simulink model "MCVT_Integration"  (R2020a)
settings: Solver=ode8  StartTime=0.0  StopTime=T_end  FixedStep=dt  RelTol=1e-3  EnableMultiTasking=on
values from neighbouring files (symbols this model uses):
  assumed_power_voltage = 1.25e2    (= 125)    [MCVT_Input_File.m]    (also in MCVT_Run_QuickCheck.m)
  battery_power_toggle = 1    [MCVT_Input_File.m]    (also in MCVT_Run_QuickCheck.m)
  battery_trickle_charge_param = 0.1    [MCVT_Input_File.m]    (also in MCVT_Run_QuickCheck.m)
  battery_trickle_leakage_param = 0.01    [MCVT_Input_File.m]    (also in MCVT_Run_QuickCheck.m)
  damage_case_id = 3    [MCVT_Input_File.m]    (also in MCVT_Run_QuickCheck.m = 1)
  dt = 1/fs    (= 0.001)    [MCVT_Run_QuickCheck.m]    (also in MCVT_Sim_Set.m)
  dt_2 = 1/fs_2    (= 0.001)    [MCVT_Run_QuickCheck.m]    (also in MCVT_Sim_Set.m)
  dt_3 = 1/fs_3    (= 0.001)    [MCVT_Run_QuickCheck.m]    (also in MCVT_Sim_Set.m)
  dt_5 = 1/fs_5    (= 0.005)    [MCVT_Run_QuickCheck.m]    (also in MCVT_Sim_Set.m)
  dt_8 = 1/fs_8    (= 0.05)    [MCVT_Run_QuickCheck.m]    (also in MCVT_Sim_Set.m)
  dt_9 = 1/fs_9    (= 0.001)    [MCVT_Run_QuickCheck.m]    (also in MCVT_Sim_Set.m)
  dust_met_duration = 3    [MCVT_Input_File.m]    (also in MCVT_Run_QuickCheck.m = 2)
  ECLSS_indoor_set_temperature = 298.15    [MCVT_Input_File.m]    (also in MCVT_Run_QuickCheck.m)
  energy_store_max_o = 150    [MCVT_Input_File.m]    (also in MCVT_Run_QuickCheck.m)
  energy_store_o = 120    [MCVT_Input_File.m]    (also in MCVT_Run_QuickCheck.m)
  fs = 1000    [MCVT_Run_QuickCheck.m]    (also in MCVT_Sim_Set.m)
  gain_charge_param = 1    [MCVT_Input_File.m]    (also in MCVT_Run_QuickCheck.m)
  ground_temperature = 373    [MCVT_Habitat_Design_Input_File.m]    (also in MCVT_Run_QuickCheck.m)
  I_max_charge = 800    [MCVT_Input_File.m]    (also in MCVT_Run_QuickCheck.m)
  I_max_discharge = 800    [MCVT_Input_File.m]    (also in MCVT_Run_QuickCheck.m)
  initial_pressure = 1e5    (= 100000)    [MCVT_Input_File.m]    (also in MCVT_Run_QuickCheck.m)
  initial_temperature = 295    [MCVT_Input_File.m]    (also in MCVT_Run_QuickCheck.m)
  interior_leak_rate = 0.00415    [MCVT_Input_File.m]    (also in MCVT_Run_QuickCheck.m)
  launch_dust_accum_start_time = 10    [MCVT_Input_File.m]    (also in MCVT_Run_QuickCheck.m = 2)
  launch_dust_accum_stop_time = 12    [MCVT_Input_File.m]    (also in MCVT_Run_QuickCheck.m = 3)
  meteorite_impact_time = 2    [MCVT_Input_File.m]    (also in MCVT_Run_QuickCheck.m = 1)
  nuclear_power_toggle = 1    [MCVT_Input_File.m]    (also in MCVT_Run_QuickCheck.m)
  print_interval = 30    [MCVT_Input_File.m]
  SOC_lower_thres = 0.5    [MCVT_Input_File.m]    (also in MCVT_Run_QuickCheck.m)
  SOC_upper_thres = 0.9    [MCVT_Input_File.m]    (also in MCVT_Run_QuickCheck.m)
  solar_power_toggle = 1    [MCVT_Input_File.m]    (also in MCVT_Run_QuickCheck.m)
  space_temperature = 4    [MCVT_Habitat_Design_Input_File.m]    (also in MCVT_Run_QuickCheck.m)
  Start_angle = 90    [MCVT_Input_File.m]    (also in MCVT_Run_QuickCheck.m)
undefined symbols (not defined in the file or in its folder): A_flow_restrictor, ACTIVITY_LOOKUP_TABLE, air, Aref, Bmap, Bmap2, Bmap3, comms, DiscIntNeverNeededParam, DmgSPL, dust_parameters, ECLSS_dust_damage_repair_time, ECLSS_heater_capacity, ECLSS_IPS_restrictor, ECLSS_IPS_setpointPressure, ECLSS_paint_damage_repair_time, ECLSS_radiatorLoop_discreteNumber, ECLSS_Vst, emptyActivity, expected_heat_add, FI, habitat, HIEM2SMM, HIEM2STM, HIEMDamageElements, impact_numbering, initial_SPLT_damage_index, input_air, input_Cond, input_CP, input_discretization, input_EV, input_Evap, input_radiatorLoop, K_ECLSS_dust_single, K_ECLSS_paint_single, K_Structure_damage_single, Kref, kW_to_W, meteoroidDustDep, mFRF, ndelays, nominalDustDep, noverlap, nuclear_toggle_gain, output_limits, panel_paint0, Ps0, reference_temperature, RepairTime_ECLSS_factor, Sensor_Location_SMM, SMM_T0, SMM_T1, SMM_T2, SMM_T3, SMM_T4, SMM_T4_0, SMM_T4_1, SMM_T4_2, solar_FDD_K ... (+73)
libraries: neural x350, simulink x39, xpcutilitieslib x7, xpcrtudplib x7, dspstat3 x1
(part 27 of 43)

== system MCVT_Integration/Sys2 - Structural System/Sys2_Structural_System/Fault Detection and Diagnosis (FDD) with Binary Classifer/Pattern Recognition Neural Network/Segment 3/Layer 2/Delays 1 ==
  "p{2}" : Inport  PortDimensions=20
      mask Display: e = 0.06;c1 = [120 230 180]/255;c2 = ([120 230 180]+0)/(256+128);c3 = ([120 230 180]+128)/(256+128);patch([0 1 1 0],[0 0 1 1],c1);patch([0 1 1 1-e 1-e 0+e],[0 0 1 1-e 0+e 0+e],c2);patch([0 0 1 1-e 0+e 0+e],[0 1 1 1-e 1-e 0+e],c3);plot([0 1 1 0 0],[0 0 1 1 0]);
  mux : Mux  Inputs=1
      mask Display: e = 0.06;c1 = [120 150 230]/255;c2 = ([120 150 230]+0)/383;c3 = ([120 150 230]+128)/383;patch([0 1 1 0],[0 0 1 1],c1);patch([0 1 1 1-e 1-e 0+e],[0 0 1 1-e 0+e 0+e],c2);patch([0 0 1 1-e 0+e 0+e],[0 1 1 1-e 1-e 0+e],c3);plot([0 1 1 0 0],[0 0 1 1 0]);disp('Mux')
  "pd{2,1}" : Outport
      mask Display: e = 0.06;c1 = [120 230 180]/255;c2 = ([120 230 180]+0)/(256+128);c3 = ([120 230 180]+128)/(256+128);patch([0 1 1 0],[0 0 1 1],c1);patch([0 1 1 1-e 1-e 0+e],[0 0 1 1-e 0+e 0+e],c2);patch([0 0 1 1-e 0+e 0+e],[0 1 1 1-e 1-e 0+e],c3);plot([0 1 1 0 0],[0 0 1 1 0]);
  wires:
  "p{2}"/1 -> mux/1
  mux/1 -> "pd{2,1}"/1

== system MCVT_Integration/Sys2 - Structural System/Sys2_Structural_System/Fault Detection and Diagnosis (FDD) with Binary Classifer/Pattern Recognition Neural Network/Segment 3/Layer 2/LW{2,1} ==
  "ad{2,1}" : Inport  PortDimensions=20
      mask Display: e = 0.06;c1 = [120 230 180]/255;c2 = ([120 230 180]+0)/(256+128);c3 = ([120 230 180]+128)/(256+128);patch([0 1 1 0],[0 0 1 1],c1);patch([0 1 1 1-e 1-e 0+e],[0 0 1 1-e 0+e 0+e],c2);patch([0 0 1 1-e 0+e 0+e],[0 1 1 1-e 1-e 0+e],c3);plot([0 1 1 0 0],[0 0 1 1 0]);
  "IW{2,1}(1,:)'" : Constant  Value=[0.15106659488629869958487006442737765610218048095703125;0.5603716392240338795005527572357095777988433837890625;-0.1423248156648591888906452140872715972363948822021484375;0.0759013429912681336642066298736608587205410003662109375;0.54039127486637228425792045527487061917781829833984375;-0.60286989011864766663251202771789394319057464599609375;-0.350069370282542535743885991905699484050273895263671875;0.321192134232399839621763248942443169653415679931640625;0.608530483384730036533483144012279808521270751953125;-0.352290986098216352129242068258463405072689056396484375;0.07942806864167507863161432624110602773725986480712890625;-0.12816484049053311622401452041231095790863037109375;-0.62042184727514015918359291390515863895416259765625;-0.0871512984286062220018465040993760339915752410888671875;-0.39129342075789674826324926470988430082798004150390625;-0.57650346467105528791563529011909849941730499267578125;-0.0189987127371317628121705212151937303133308887481689453125;-0.052366305100728267885035194240117562003433704376220703125;-0.12236912546824448011495434229800594039261341094970703125;-0.1765566654176617955585015806718729436397552490234375]
      mask Display: e = 0.06;c1 = [120 150 230]/255;c2 = ([120 150 230]+0)/383;c3 = ([120 150 230]+128)/383;patch([0 1 1 0],[0 0 1 1],c1);patch([0 1 1 1-e 1-e 0+e],[0 0 1 1-e 0+e 0+e],c2);patch([0 0 1 1-e 0+e 0+e],[0 1 1 1-e 1-e 0+e],c3);plot([0 1 1 0 0],[0 0 1 1 0]);disp('weights');
  "IW{2,1}(10,:)'" : Constant  Value=[0.2291636645137108130310110709615400992333889007568359375;-0.4266525376677356984345124146784655749797821044921875;0.50903179686960131622441849685856141149997711181640625;-0.421901876015020549370859725968330167233943939208984375;-0.12078580318779984226207346864612190984189510345458984375;0.487182207938093914112442917030421085655689239501953125;-0.5630564938925262108426750273793004453182220458984375;-0.1495304627572677791391697610379196703433990478515625;-0.1695783473312574562985588499941513873636722564697265625;0.4017534498995158909195879459730349481105804443359375;0.283421176761598225812832652081851847469806671142578125;0.0688854705714498638524645457437145523726940155029296875;0.2320481338533733894902155725503689609467983245849609375;0.4621838504146518200599302872433327138423919677734375;-0.43578605399504521944464840998989529907703399658203125;-0.2105588758046655628586307784644304774701595306396484375;-0.397997367611341579252126621213392354547977447509765625;-0.38610469116421086699375564421643503010272979736328125;-0.36761991533750559835169724465231411159038543701171875;0.534979662095591113057935217511840164661407470703125]
      mask Display: e = 0.06;c1 = [120 150 230]/255;c2 = ([120 150 230]+0)/383;c3 = ([120 150 230]+128)/383;patch([0 1 1 0],[0 0 1 1],c1);patch([0 1 1 1-e 1-e 0+e],[0 0 1 1-e 0+e 0+e],c2);patch([0 0 1 1-e 0+e 0+e],[0 1 1 1-e 1-e 0+e],c3);plot([0 1 1 0 0],[0 0 1 1 0]);disp('weights');
  "IW{2,1}(11,:)'" : Constant  Value=[-0.5081275684215835752866041730158030986785888671875;0.1073158041467901224752523603456211276352405548095703125;-0.07361132813026703691772212323485291562974452972412109375;0.450550089877159665174843894419609569013118743896484375;0.0086593430160507821324866739587378106079995632171630859375;0.4037706239645844075170089126913808286190032958984375;0.066226835544588968929247130290605127811431884765625;-0.47846038642917310124147434180486015975475311279296875;-0.5655856381792616627279812746564857661724090576171875;0.323335764149915771970000832880032248795032501220703125;-0.191630875086526486494875598509679548442363739013671875;-0.25212709054856052848236913632717914879322052001953125;0.514191784319361744337584241293370723724365234375;0.43787936653556525090635886954260058701038360595703125;-0.466271158413999298186780606556567363440990447998046875;-0.2492973956702883298053308180897147394716739654541015625;0.60419760698692581701862991394591517746448516845703125;-0.3321250203051395732956052597728557884693145751953125;0.195539095225074677220078456230112351477146148681640625;0.003284635648635132743056175286255893297493457794189453125]
      mask Display: e = 0.06;c1 = [120 150 230]/255;c2 = ([120 150 230]+0)/383;c3 = ([120 150 230]+128)/383;patch([0 1 1 0],[0 0 1 1],c1);patch([0 1 1 1-e 1-e 0+e],[0 0 1 1-e 0+e 0+e],c2);patch([0 0 1 1-e 0+e 0+e],[0 1 1 1-e 1-e 0+e],c3);plot([0 1 1 0 0],[0 0 1 1 0]);disp('weights');
  "IW{2,1}(12,:)'" : Constant  Value=[0.119778454764274899790876816041418351233005523681640625;0.26307119451012550559454439280671067535877227783203125;0.2098396983730937692680384998311637900769710540771484375;0.263145661726699564564313504888559691607952117919921875;-0.1686105570722050417398207855512737296521663665771484375;0.27524520078677239265374510068795643746852874755859375;-0.374470993919618522216552491954644210636615753173828125;-0.5218050287758202454568845496396534144878387451171875;-0.28876775132229359766000698073185048997402191162109375;0.430728407233508858720227863159379921853542327880859375;0.408571982210275574942670573364011943340301513671875;0.57259534482969132174190463047125376760959625244140625;-0.300792436945121810509817805723287165164947509765625;-0.34842733554069049706214400430326350033283233642578125;-0.410345962419400300813521198506350629031658172607421875;0.2408701059818025569381205741592566482722759246826171875;-0.49539967877842949750544221387826837599277496337890625;-0.58939302896003697984639302376308478415012359619140625;0.361398950573516675177643264760263264179229736328125;0.057440338599546392206462996909976936876773834228515625]
      mask Display: e = 0.06;c1 = [120 150 230]/255;c2 = ([120 150 230]+0)/383;c3 = ([120 150 230]+128)/383;patch([0 1 1 0],[0 0 1 1],c1);patch([0 1 1 1-e 1-e 0+e],[0 0 1 1-e 0+e 0+e],c2);patch([0 0 1 1-e 0+e 0+e],[0 1 1 1-e 1-e 0+e],c3);plot([0 1 1 0 0],[0 0 1 1 0]);disp('weights');
  "IW{2,1}(13,:)'" : Constant  Value=[-0.441722096799943175771119285855093039572238922119140625;0.16235866190285619392597027399460785090923309326171875;0.0979013490574758282747325210948474705219268798828125;0.466997659864763436932122431244351901113986968994140625;0.186051815556723620925794193681213073432445526123046875;-0.25975999000550320516822466743178665637969970703125;0.54121175498833895556316520014661364257335662841796875;-0.140309970646500747903928640880621969699859619140625;0.396614050852546196068715289584361016750335693359375;0.355946059060724462330682626998168416321277618408203125;-0.11637527029470472184069507193271419964730739593505859375;-0.1935716655116017370641401385000790469348430633544921875;-0.5343577089758266840391343066585250198841094970703125;0.48468449111628297831799727646284736692905426025390625;0.3977550528000162177733045609784312546253204345703125;0.58906810595575109790189571867813356220722198486328125;-0.417009003018671275953010990633629262447357177734375;0.115480344774782028860471427833545021712779998779296875;-0.487311372596715919325305321763153187930583953857421875;-0.2218206660506606142657659574979334138333797454833984375]
      mask Display: e = 0.06;c1 = [120 150 230]/255;c2 = ([120 150 230]+0)/383;c3 = ([120 150 230]+128)/383;patch([0 1 1 0],[0 0 1 1],c1);patch([0 1 1 1-e 1-e 0+e],[0 0 1 1-e 0+e 0+e],c2);patch([0 0 1 1-e 0+e 0+e],[0 1 1 1-e 1-e 0+e],c3);plot([0 1 1 0 0],[0 0 1 1 0]);disp('weights');
  "IW{2,1}(14,:)'" : Constant  Value=[0.334252666560719224886355505077517591416835784912109375;0.1983137030024247071668952457912382669746875762939453125;0.319917486701020326034239360524225048720836639404296875;0.514369637551277403275662436499260365962982177734375;0.41893065578403732018841765238903462886810302734375;-0.52696353696210762596052745720953680574893951416015625;0.32334424598154054564247417147271335124969482421875;0.1444979041196569335969712710721069015562534332275390625;-0.59017262538534331905992758038337342441082000732421875;-0.071897903013939779004459751376998610794544219970703125;-0.5185410461693258366011605176026932895183563232421875;0.039306984091826181748619006839362555183470249176025390625;-0.138762770826605985607926641023368574678897857666015625;0.244754399416360290953065259600407443940639495849609375;0.59865502790181068792918495091726072132587432861328125;0.2495828864728054374833021711310721002519130706787109375;0.2280241535022842214175398112274706363677978515625;0.258109283529607769214209156416472978889942169189453125;0.398036230457980588770539043252938427031040191650390625;-0.395826804041779334308870375025435350835323333740234375]
      mask Display: e = 0.06;c1 = [120 150 230]/255;c2 = ([120 150 230]+0)/383;c3 = ([120 150 230]+128)/383;patch([0 1 1 0],[0 0 1 1],c1);patch([0 1 1 1-e 1-e 0+e],[0 0 1 1-e 0+e 0+e],c2);patch([0 0 1 1-e 0+e 0+e],[0 1 1 1-e 1-e 0+e],c3);plot([0 1 1 0 0],[0 0 1 1 0]);disp('weights');
  "IW{2,1}(15,:)'" : Constant  Value=[-0.556975696474056203300051492988131940364837646484375;-0.13936353715497429295311349051189608871936798095703125;-0.140929639782798654668027893421822227537631988525390625;-0.187891128628529913147104934978415258228778839111328125;-0.09801446602994483281445781130969407968223094940185546875;-0.496058295664981108163971157409832812845706939697265625;0.272751909979205786616063278415822423994541168212890625;0.049045669396399482753423626490985043346881866455078125;-0.319617920831514246149396285545662976801395416259765625;-0.0817837639424334461590859746138448826968669891357421875;-0.46758310253976087533800409801187925040721893310546875;0.2299988954843138200256902337059727869927883148193359375;0.59455168739725172688537213616655208170413970947265625;-0.21281710823811017530005074149812571704387664794921875;0.660491621708700904491706751286983489990234375;0.241771012644863336760181482532061636447906494140625;-0.42505740111873624442750951857306063175201416015625;0.418160610199512061679882890530279837548732757568359375;0.221425335063371198085491187157458625733852386474609375;0.51483980059742029400382534731761552393436431884765625]
      mask Display: e = 0.06;c1 = [120 150 230]/255;c2 = ([120 150 230]+0)/383;c3 = ([120 150 230]+128)/383;patch([0 1 1 0],[0 0 1 1],c1);patch([0 1 1 1-e 1-e 0+e],[0 0 1 1-e 0+e 0+e],c2);patch([0 0 1 1-e 0+e 0+e],[0 1 1 1-e 1-e 0+e],c3);plot([0 1 1 0 0],[0 0 1 1 0]);disp('weights');
  "IW{2,1}(16,:)'" : Constant  Value=[0.001448849258909091532177004779669005074538290500640869140625;-0.08077289923198492649358826156458235345780849456787109375;-0.2439634430585133628710536868311464786529541015625;-0.4293216611635575219452221062965691089630126953125;0.2830568458138678078483962963218800723552703857421875;0.2185972831924547776782929986438830383121967315673828125;0.273020106171740828404637113635544665157794952392578125;-0.72230733881549025188206769598764367401599884033203125;0.1180729273415092872578924243498477153480052947998046875;0.0990293151879542421500701721015502698719501495361328125;0.5789329217545320371840489315218292176723480224609375;-0.278249205078244743472026812014519236981868743896484375;0.59460975877889066243398019651067443192005157470703125;0.014227279816829023484370253527231398038566112518310546875;-0.3488117237190468955532196559943258762359619140625;0.16175346286436009801690261156181804835796356201171875;0.006201872917077967152510442616630825796164572238922119140625;-0.7118344437533761226433171032113023102283477783203125;-0.0702931236980166296302030559672857634723186492919921875;-0.60628695408514665654564623764599673449993133544921875]
      mask Display: e = 0.06;c1 = [120 150 230]/255;c2 = ([120 150 230]+0)/383;c3 = ([120 150 230]+128)/383;patch([0 1 1 0],[0 0 1 1],c1);patch([0 1 1 1-e 1-e 0+e],[0 0 1 1-e 0+e 0+e],c2);patch([0 0 1 1-e 0+e 0+e],[0 1 1 1-e 1-e 0+e],c3);plot([0 1 1 0 0],[0 0 1 1 0]);disp('weights');
  "IW{2,1}(17,:)'" : Constant  Value=[0.142705906197661491763284402622957713901996612548828125;-0.34671194441398045515967396568157710134983062744140625;-0.182315930820515614829702144561451859772205352783203125;-0.5980011997163632297969115825253538787364959716796875;0.2020122842986547817556441941633238457143306732177734375;0.09644959216750241537408072645121137611567974090576171875;0.07045050718211458284923054407045128755271434783935546875;0.06909960768209812143059167510728002525866031646728515625;0.206588882691997988150234277782146818935871124267578125;-0.322269682554155501019721441480214707553386688232421875;0.6213591289461550815076407161541283130645751953125;0.372396006410809388142979514668695628643035888671875;0.440626866276933537580617894491297192871570587158203125;-0.34991724067220408844747225884930230677127838134765625;0.389120287318546420607390246004797518253326416015625;0.52924523174505810896306456925231032073497772216796875;-0.25869389758506800003345915683894418179988861083984375;0.46277250516199053276267250112141482532024383544921875;-0.16545831196350047065379840205423533916473388671875;-0.58245908001027502098878585456986911594867706298828125]
      mask Display: e = 0.06;c1 = [120 150 230]/255;c2 = ([120 150 230]+0)/383;c3 = ([120 150 230]+128)/383;patch([0 1 1 0],[0 0 1 1],c1);patch([0 1 1 1-e 1-e 0+e],[0 0 1 1-e 0+e 0+e],c2);patch([0 0 1 1-e 0+e 0+e],[0 1 1 1-e 1-e 0+e],c3);plot([0 1 1 0 0],[0 0 1 1 0]);disp('weights');
  "IW{2,1}(18,:)'" : Constant  Value=[-0.0960541113227868226243089111449080519378185272216796875;0.65946213787547736107086393531062640249729156494140625;0.11548970165775103435823467634691041894257068634033203125;-0.45966530423970730634408710102434270083904266357421875;-0.66569293690471320612545014228089712560176849365234375;-0.382446206821509260276314989823731593787670135498046875;0.15723445705206129385800295494846068322658538818359375;0.443993078480637459737323524677776731550693511962890625;0.029827736818623641623648978793426067568361759185791015625;-0.021938976143679463082225566950000938959419727325439453125;-0.25206662362630261053908498070086352527141571044921875;0.46433140045529441852778518295963294804096221923828125;-0.02119412796122412123711598042063997127115726470947265625;-0.293965828500245607290963789637316949665546417236328125;-0.16392863557610504177119992164080031216144561767578125;0.63431763291851017694256142931408248841762542724609375;-0.207311398098050769789324476732872426509857177734375;-0.4390693592890702046105388944852165877819061279296875;-0.394522288710088153695920709651545621454715728759765625;0.07119679106039593363686179827709565870463848114013671875]
      mask Display: e = 0.06;c1 = [120 150 230]/255;c2 = ([120 150 230]+0)/383;c3 = ([120 150 230]+128)/383;patch([0 1 1 0],[0 0 1 1],c1);patch([0 1 1 1-e 1-e 0+e],[0 0 1 1-e 0+e 0+e],c2);patch([0 0 1 1-e 0+e 0+e],[0 1 1 1-e 1-e 0+e],c3);plot([0 1 1 0 0],[0 0 1 1 0]);disp('weights');
  "IW{2,1}(19,:)'" : Constant  Value=[-0.39776934077347181339945336731034331023693084716796875;-0.5186691691131677384163367605651728808879852294921875;0.59564415540282300565166906380909495055675506591796875;0.5110679886000990546079947307589463889598846435546875;-0.1940612014466223111952558610937558114528656005859375;0.528959291659615526981497168890200555324554443359375;0.357640373204124062400666161920526064932346343994140625;0.324125279308876079742418596651987172663211822509765625;-0.016669069698846690485982691143362899310886859893798828125;-0.125756942302969354496866571935242973268032073974609375;0.4267477914157584706345005542971193790435791015625;-0.2344278005652788987589474345440976321697235107421875;0.09144978984671647825877016657614149153232574462890625;-0.267397494402711821681606352285598404705524444580078125;0.2342586603755367480328430929148453287780284881591796875;-0.53299716414215325688275015636463649570941925048828125;-0.29794780195952019763439011512673459947109222412109375;-0.31572630007257107553897412799415178596973419189453125;-0.367248638764981816251520285732112824916839599609375;-0.220506357540645792081335230250260792672634124755859375]
      mask Display: e = 0.06;c1 = [120 150 230]/255;c2 = ([120 150 230]+0)/383;c3 = ([120 150 230]+128)/383;patch([0 1 1 0],[0 0 1 1],c1);patch([0 1 1 1-e 1-e 0+e],[0 0 1 1-e 0+e 0+e],c2);patch([0 0 1 1-e 0+e 0+e],[0 1 1 1-e 1-e 0+e],c3);plot([0 1 1 0 0],[0 0 1 1 0]);disp('weights');
  "IW{2,1}(2,:)'" : Constant  Value=[0.288109672434351427039445070477086119353771209716796875;-0.41070083022694492402848709389218129217624664306640625;0.0411054074150142556565157292425283230841159820556640625;-0.2893980942947376178153717773966491222381591796875;-0.51834467401478334647180190586368553340435028076171875;-0.52103588836250225302393346282769925892353057861328125;0.41094187933909565035861533033312298357486724853515625;0.353327781787153849535343397292308509349822998046875;-0.1560737375214712729931676449268707074224948883056640625;0.241145063602334397412363387047662399709224700927734375;-0.50503101238212677603911515689105726778507232666015625;0.251368985022243196514324381496408022940158843994140625;0.049613543042057568766001196536308270879089832305908203125;-0.59081236526510638729092761423089541494846343994140625;-0.484430474687280787637888579411082901060581207275390625;-0.34238364817464661538082282277173362672328948974609375;-0.11957700273052761230641038991961977444589138031005859375;-0.251904161351189426198970977566204965114593505859375;-0.422015888742625577823019966672291047871112823486328125;0.36497146629934584804999531115754507482051849365234375]
      mask Display: e = 0.06;c1 = [120 150 230]/255;c2 = ([120 150 230]+0)/383;c3 = ([120 150 230]+128)/383;patch([0 1 1 0],[0 0 1 1],c1);patch([0 1 1 1-e 1-e 0+e],[0 0 1 1-e 0+e 0+e],c2);patch([0 0 1 1-e 0+e 0+e],[0 1 1 1-e 1-e 0+e],c3);plot([0 1 1 0 0],[0 0 1 1 0]);disp('weights');
  "IW{2,1}(20,:)'" : Constant  Value=[-0.06522277116647141881333737956083496101200580596923828125;0.1933112015870522004679088468037662096321582794189453125;-0.416586844632332919946549054657225497066974639892578125;0.65131643998191623978755160351283848285675048828125;0.54420129448868415078521820760215632617473602294921875;-0.12307449612004321692904085239206324331462383270263671875;-0.361778952422099120411758121917955577373504638671875;0.11630697053673812180196733834236511029303073883056640625;0.300505373693401522938728476219694130122661590576171875;-0.378920023141883610673374960242654196918010711669921875;0.0156781624133405204923885634116231813095510005950927734375;0.50279279919684682909064576961100101470947265625;0.1441228538065199848983155561654712073504924774169921875;0.2407901982226343939874624311414663679897785186767578125;0.07983633444273295631798958993385895155370235443115234375;0.1355979782335982875007829306923667900264263153076171875;0.5390974029418338364649798677419312298297882080078125;-0.51989969646466460684308685813448391854763031005859375;-0.6428061801909652483999479954945854842662811279296875;0.11129032883898780370568459829883067868649959564208984375]
      mask Display: e = 0.06;c1 = [120 150 230]/255;c2 = ([120 150 230]+0)/383;c3 = ([120 150 230]+128)/383;patch([0 1 1 0],[0 0 1 1],c1);patch([0 1 1 1-e 1-e 0+e],[0 0 1 1-e 0+e 0+e],c2);patch([0 0 1 1-e 0+e 0+e],[0 1 1 1-e 1-e 0+e],c3);plot([0 1 1 0 0],[0 0 1 1 0]);disp('weights');
  "IW{2,1}(3,:)'" : Constant  Value=[0.2059551799348844358661381193087436258792877197265625;0.310047661201032942468458486473537050187587738037109375;0.4770412414927973632217117483378387987613677978515625;-0.385557646241332874215146375718177296221256256103515625;-0.1946628047623883694949853406797046773135662078857421875;-0.10621822597456349879241344069669139571487903594970703125;-0.54848621495900784506005720686516724526882171630859375;-0.247482022027998904700751836571726016700267791748046875;-0.037810811052910335561261234715857426635921001434326171875;-0.2415011780421596199186495823596487753093242645263671875;0.477590076061509327676191105638281442224979400634765625;0.381449911823697973556335227840463630855083465576171875;-0.56111061463163969253997720443294383585453033447265625;-0.273248307467886031307813254898064769804477691650390625;-0.49619804346761131608189998587477020919322967529296875;0.431057256052138126900530323837301693856716156005859375;0.200466204042757667469487614653189666569232940673828125;0.60072233036356903479457969297072850167751312255859375;-0.270181911302637967065010116129997186362743377685546875;-0.12387073829872501107285387433876167051494121551513671875]
      mask Display: e = 0.06;c1 = [120 150 230]/255;c2 = ([120 150 230]+0)/383;c3 = ([120 150 230]+128)/383;patch([0 1 1 0],[0 0 1 1],c1);patch([0 1 1 1-e 1-e 0+e],[0 0 1 1-e 0+e 0+e],c2);patch([0 0 1 1-e 0+e 0+e],[0 1 1 1-e 1-e 0+e],c3);plot([0 1 1 0 0],[0 0 1 1 0]);disp('weights');
  "IW{2,1}(4,:)'" : Constant  Value=[0.2161661880723786677283015933426213450729846954345703125;-0.1833528114950019050066742920535034500062465667724609375;0.1916055155418400490763275456629344262182712554931640625;0.01778854457812235889679897127280128188431262969970703125;-0.134444769234163263860182269127108156681060791015625;0.375438024362276523415715701048611663281917572021484375;-0.1668065926176147961701445865401183255016803741455078125;0.52005801907834736663716057591955177485942840576171875;-0.408614574848783440597088656431878916919231414794921875;-0.5544565168419239853392355144023895263671875;0.71468849431567649244101403382956050336360931396484375;0.421376111739312719794980921506066806614398956298828125;0.2408921921068725990888736987471929751336574554443359375;-0.64810596621699823050022359893773682415485382080078125;0.39134302229348982837819903579656966030597686767578125;-0.367748944126091992234961480789934284985065460205078125;-0.1636543035435838178148770793995936401188373565673828125;-0.09049030261419953313772879255338921211659908294677734375;0.1846779356533845284360495497821830213069915771484375;0.267251941491772504466695181690738536417484283447265625]
      mask Display: e = 0.06;c1 = [120 150 230]/255;c2 = ([120 150 230]+0)/383;c3 = ([120 150 230]+128)/383;patch([0 1 1 0],[0 0 1 1],c1);patch([0 1 1 1-e 1-e 0+e],[0 0 1 1-e 0+e 0+e],c2);patch([0 0 1 1-e 0+e 0+e],[0 1 1 1-e 1-e 0+e],c3);plot([0 1 1 0 0],[0 0 1 1 0]);disp('weights');
  "IW{2,1}(5,:)'" : Constant  Value=[0.25989642851030969605830023283488117158412933349609375;0.1760892576795972386261013298280886374413967132568359375;0.61528983202339759817078856940497644245624542236328125;0.1475772770292280433590548227584804408252239227294921875;-0.26524867147067843209384818692342378199100494384765625;0.3999764393541369589257783445646055042743682861328125;-0.166364709218893958020402124020620249211788177490234375;0.1277838893056524038893684291906538419425487518310546875;0.635659231019399584283746662549674510955810546875;0.291613850537881413860219481648528017103672027587890625;-0.25718912070789212709343019014340825378894805908203125;-0.53569769371968367632774743469781242311000823974609375;-0.061948602546908503041489524321150383912026882171630859375;0.2197035171515357709903781824323232285678386688232421875;0.5621349107808291112320375759736634790897369384765625;-0.1770781902813020336484584049685508944094181060791015625;-0.64425935334126982478863965297932736575603485107421875;-0.1466150322245029780088287907346966676414012908935546875;-0.32357940201068802199557694621034897863864898681640625;0.30803804600077933084634196347906254231929779052734375]
      mask Display: e = 0.06;c1 = [120 150 230]/255;c2 = ([120 150 230]+0)/383;c3 = ([120 150 230]+128)/383;patch([0 1 1 0],[0 0 1 1],c1);patch([0 1 1 1-e 1-e 0+e],[0 0 1 1-e 0+e 0+e],c2);patch([0 0 1 1-e 0+e 0+e],[0 1 1 1-e 1-e 0+e],c3);plot([0 1 1 0 0],[0 0 1 1 0]);disp('weights');
  "IW{2,1}(6,:)'" : Constant  Value=[0.50180470250115105645960511537850834429264068603515625;-0.000915630053442912056164970646676692922483198344707489013671875;0.406773676269099715430144215133623220026493072509765625;0.01289726795588202155895540812480248860083520412445068359375;0.044081127189506019714571039003203622996807098388671875;0.07227793888596416305158953719001146964728832244873046875;-0.1141843252420678134217979504683171398937702178955078125;0.58811855841279914169916764876688830554485321044921875;-0.477410433738430117767137517148512415587902069091796875;0.1309929211810685056871506048992159776389598846435546875;-0.0265292107416204257785974363059722236357629299163818359375;0.392392948284501608124941185451461933553218841552734375;-0.0165044125151770047776889072110861889086663722991943359375;0.63718374493217233567321500231628306210041046142578125;0.636314445894762048538950693910010159015655517578125;-0.4836862713794138901590713430778123438358306884765625;-0.27434177268281978445685354017768986523151397705078125;0.0937938277016527044427363080103532411158084869384765625;0.46291011938007786685744804344722069799900054931640625;0.386709536538152154339087473999825306236743927001953125]
      mask Display: e = 0.06;c1 = [120 150 230]/255;c2 = ([120 150 230]+0)/383;c3 = ([120 150 230]+128)/383;patch([0 1 1 0],[0 0 1 1],c1);patch([0 1 1 1-e 1-e 0+e],[0 0 1 1-e 0+e 0+e],c2);patch([0 0 1 1-e 0+e 0+e],[0 1 1 1-e 1-e 0+e],c3);plot([0 1 1 0 0],[0 0 1 1 0]);disp('weights');
  "IW{2,1}(7,:)'" : Constant  Value=[0.04934571371614958346096813102121814154088497161865234375;-0.3669002529098606135704585540224798023700714111328125;0.50980856454774059471901637152768671512603759765625;-0.1268478657659717068195703859601053409278392791748046875;0.138773250533559544539485841596615500748157501220703125;-0.06524253855661142631472415587268187664449214935302734375;0.2254949265086063914242942018972826190292835235595703125;0.5816268480267721141530046224943362176418304443359375;0.51421465220765127934754445959697477519512176513671875;0.43023425861352404542259364461642690002918243408203125;-0.331457286536438189283870769941131584346294403076171875;0.46037893886767655704517210324411280453205108642578125;0.165566455070217888323469423994538374245166778564453125;0.486870147485172599655101066673523746430873870849609375;0.110372869831219355685192340388312004506587982177734375;-0.52139681288416728222046003793366253376007080078125;-0.1763470456458597623861095371466944925487041473388671875;-0.5329577924538166922019399862620048224925994873046875;-0.32039921856353192897159942731377668678760528564453125;-0.315281737108870474539656925117014907300472259521484375]
      mask Display: e = 0.06;c1 = [120 150 230]/255;c2 = ([120 150 230]+0)/383;c3 = ([120 150 230]+128)/383;patch([0 1 1 0],[0 0 1 1],c1);patch([0 1 1 1-e 1-e 0+e],[0 0 1 1-e 0+e 0+e],c2);patch([0 0 1 1-e 0+e 0+e],[0 1 1 1-e 1-e 0+e],c3);plot([0 1 1 0 0],[0 0 1 1 0]);disp('weights');
  "IW{2,1}(8,:)'" : Constant  Value=[0.395147555648888426649278926561237312853336334228515625;0.32450001981837706122036024680710397660732269287109375;-0.299180136532453622155713901520357467234134674072265625;-0.2343122849635475291041331047381390817463397979736328125;0.371736845433375096003913995446055196225643157958984375;-0.2020868742875994161778407942620106041431427001953125;0.157804417997004453599885209769126959145069122314453125;-0.05025227752920123636126703559057204984128475189208984375;-0.52743386853732376895464994959183968603610992431640625;-0.292552248398340319912591667161905206739902496337890625;-0.417732261637839108470160454089636914432048797607421875;0.4086736385739657695381765734055079519748687744140625;-0.48016653454358471098117888686829246580600738525390625;0.52679404676409047336704816189012490212917327880859375;0.6022855883237518970219070979510433971881866455078125;-0.58469907257618436435819830876425839960575103759765625;-0.0039964584862391128172731669110362417995929718017578125;-0.2128582965766855072953234184751636348664760589599609375;0.1204021223952279895375028218040824867784976959228515625;0.22896059354461473223096845686086453497409820556640625]
      mask Display: e = 0.06;c1 = [120 150 230]/255;c2 = ([120 150 230]+0)/383;c3 = ([120 150 230]+128)/383;patch([0 1 1 0],[0 0 1 1],c1);patch([0 1 1 1-e 1-e 0+e],[0 0 1 1-e 0+e 0+e],c2);patch([0 0 1 1-e 0+e 0+e],[0 1 1 1-e 1-e 0+e],c3);plot([0 1 1 0 0],[0 0 1 1 0]);disp('weights');
  "IW{2,1}(9,:)'" : Constant  Value=[-0.38508834307233097415945621833088807761669158935546875;-0.478166060542723092563477393923676572740077972412109375;-0.081486776888633094539926560173626057803630828857421875;-0.39784877834228449255959958463790826499462127685546875;0.52095525837487477449627704118029214441776275634765625;-0.0222252435208852017201053286044043488800525665283203125;0.60327819751150635330105842513148672878742218017578125;-0.5546996197314240095721515899640507996082305908203125;0.03573876066296789721921101090629235841333866119384765625;-0.4717216392592902263203313850681297481060028076171875;-0.12851474552296038300625014016986824572086334228515625;-0.144309823892515620702425849231076426804065704345703125;0.359765513053050323133419396981480531394481658935546875;0.291749660666982879586583976561087183654308319091796875;-0.119774491580091346776271166163496673107147216796875;-0.40193574733741777027518082832102663815021514892578125;-0.239008414564039262106831529308692552149295806884765625;-0.385718234824624028878048420665436424314975738525390625;0.476237654884348937667226664416375569999217987060546875;0.460723172613386022788972695707343518733978271484375]
      mask Display: e = 0.06;c1 = [120 150 230]/255;c2 = ([120 150 230]+0)/383;c3 = ([120 150 230]+128)/383;patch([0 1 1 0],[0 0 1 1],c1);patch([0 1 1 1-e 1-e 0+e],[0 0 1 1-e 0+e 0+e],c2);patch([0 0 1 1-e 0+e 0+e],[0 1 1 1-e 1-e 0+e],c3);plot([0 1 1 0 0],[0 0 1 1 0]);disp('weights');
  Mux : Mux  Inputs=20
      mask Display: e = 0.06;c1 = [120 150 230]/255;c2 = ([120 150 230]+0)/383;c3 = ([120 150 230]+128)/383;patch([0 1 1 0],[0 0 1 1],c1);patch([0 1 1 1-e 1-e 0+e],[0 0 1 1-e 0+e 0+e],c2);patch([0 0 1 1-e 0+e 0+e],[0 1 1 1-e 1-e 0+e],c3);plot([0 1 1 0 0],[0 0 1 1 0]);disp('Mux');
  dotprod{1..20} (20 alike) : neural/Weight Functions/dotprod
  "lz{2,1}" : Outport
      mask Display: e = 0.06;c1 = [120 230 180]/255;c2 = ([120 230 180]+0)/(256+128);c3 = ([120 230 180]+128)/(256+128);patch([0 1 1 0],[0 0 1 1],c1);patch([0 1 1 1-e 1-e 0+e],[0 0 1 1-e 0+e 0+e],c2);patch([0 0 1 1-e 0+e 0+e],[0 1 1 1-e 1-e 0+e],c3);plot([0 1 1 0 0],[0 0 1 1 0]);
  wires:
  "IW{2,1}(1,:)'"/1 -> dotprod1/1
  "ad{2,1}"/1 -> dotprod1/2, dotprod2/2, dotprod3/2, dotprod4/2, dotprod5/2, dotprod6/2, dotprod7/2, dotprod8/2, dotprod9/2, dotprod10/2, dotprod11/2, dotprod12/2, dotprod13/2, dotprod14/2, dotprod15/2, dotprod16/2, dotprod17/2, dotprod18/2, dotprod19/2, dotprod20/2
  dotprod1/1 -> Mux/1
  "IW{2,1}(2,:)'"/1 -> dotprod2/1
  dotprod2/1 -> Mux/2
  "IW{2,1}(3,:)'"/1 -> dotprod3/1
  dotprod3/1 -> Mux/3
  "IW{2,1}(4,:)'"/1 -> dotprod4/1
  dotprod4/1 -> Mux/4
  "IW{2,1}(5,:)'"/1 -> dotprod5/1
  dotprod5/1 -> Mux/5
  "IW{2,1}(6,:)'"/1 -> dotprod6/1
  dotprod6/1 -> Mux/6
  "IW{2,1}(7,:)'"/1 -> dotprod7/1
  dotprod7/1 -> Mux/7
  "IW{2,1}(8,:)'"/1 -> dotprod8/1
  dotprod8/1 -> Mux/8
  "IW{2,1}(9,:)'"/1 -> dotprod9/1
  dotprod9/1 -> Mux/9
  "IW{2,1}(10,:)'"/1 -> dotprod10/1
  dotprod10/1 -> Mux/10
  "IW{2,1}(11,:)'"/1 -> dotprod11/1
  dotprod11/1 -> Mux/11
  "IW{2,1}(12,:)'"/1 -> dotprod12/1
  dotprod12/1 -> Mux/12
  "IW{2,1}(13,:)'"/1 -> dotprod13/1
  dotprod13/1 -> Mux/13
  "IW{2,1}(14,:)'"/1 -> dotprod14/1
  dotprod14/1 -> Mux/14
  "IW{2,1}(15,:)'"/1 -> dotprod15/1
  dotprod15/1 -> Mux/15
  "IW{2,1}(16,:)'"/1 -> dotprod16/1
  dotprod16/1 -> Mux/16
  "IW{2,1}(17,:)'"/1 -> dotprod17/1
  dotprod17/1 -> Mux/17
  "IW{2,1}(18,:)'"/1 -> dotprod18/1
  dotprod18/1 -> Mux/18
  "IW{2,1}(19,:)'"/1 -> dotprod19/1
  dotprod19/1 -> Mux/19
  "IW{2,1}(20,:)'"/1 -> dotprod20/1
  dotprod20/1 -> Mux/20
  Mux/1 -> "lz{2,1}"/1

== system MCVT_Integration/Sys2 - Structural System/Sys2_Structural_System/Fault Detection and Diagnosis (FDD) with Binary Classifer/Pattern Recognition Neural Network/Segment 3/Layer 3/Delays 1 ==
  "p{3}" : Inport  PortDimensions=20
      mask Display: e = 0.06;c1 = [120 230 180]/255;c2 = ([120 230 180]+0)/(256+128);c3 = ([120 230 180]+128)/(256+128);patch([0 1 1 0],[0 0 1 1],c1);patch([0 1 1 1-e 1-e 0+e],[0 0 1 1-e 0+e 0+e],c2);patch([0 0 1 1-e 0+e 0+e],[0 1 1 1-e 1-e 0+e],c3);plot([0 1 1 0 0],[0 0 1 1 0]);
  mux : Mux  Inputs=1
      mask Display: e = 0.06;c1 = [120 150 230]/255;c2 = ([120 150 230]+0)/383;c3 = ([120 150 230]+128)/383;patch([0 1 1 0],[0 0 1 1],c1);patch([0 1 1 1-e 1-e 0+e],[0 0 1 1-e 0+e 0+e],c2);patch([0 0 1 1-e 0+e 0+e],[0 1 1 1-e 1-e 0+e],c3);plot([0 1 1 0 0],[0 0 1 1 0]);disp('Mux')
  "pd{3,2}" : Outport
      mask Display: e = 0.06;c1 = [120 230 180]/255;c2 = ([120 230 180]+0)/(256+128);c3 = ([120 230 180]+128)/(256+128);patch([0 1 1 0],[0 0 1 1],c1);patch([0 1 1 1-e 1-e 0+e],[0 0 1 1-e 0+e 0+e],c2);patch([0 0 1 1-e 0+e 0+e],[0 1 1 1-e 1-e 0+e],c3);plot([0 1 1 0 0],[0 0 1 1 0]);
  wires:
  "p{3}"/1 -> mux/1
  mux/1 -> "pd{3,2}"/1

== system MCVT_Integration/Sys2 - Structural System/Sys2_Structural_System/Fault Detection and Diagnosis (FDD) with Binary Classifer/Pattern Recognition Neural Network/Segment 3/Layer 3/LW{3,2} ==
  "ad{3,2}" : Inport  PortDimensions=20
      mask Display: e = 0.06;c1 = [120 230 180]/255;c2 = ([120 230 180]+0)/(256+128);c3 = ([120 230 180]+128)/(256+128);patch([0 1 1 0],[0 0 1 1],c1);patch([0 1 1 1-e 1-e 0+e],[0 0 1 1-e 0+e 0+e],c2);patch([0 0 1 1-e 0+e 0+e],[0 1 1 1-e 1-e 0+e],c3);plot([0 1 1 0 0],[0 0 1 1 0]);
  "IW{3,2}(1,:)'" : Constant  Value=[0.63648340515791590465966010015108622610569000244140625;0.54720498059730104944975437319953925907611846923828125;-0.28071013847852988032371968074585311114788055419921875;-0.63213757400478065573423691603238694369792938232421875;-0.49347224452879145939476757121155969798564910888671875;0.84046066972557564866974644246511161327362060546875;0.71810707526801798028515122496173717081546783447265625;0.70421483520518501908469488625996746122837066650390625;-0.229471447354115343220115619260468520224094390869140625;0.8664653171316583080141526806983165442943572998046875;0.252741460775858406417881951711024157702922821044921875;0.6561257517779370829202889581210911273956298828125;-0.94757521768273422768658065251656807959079742431640625;0.79813549261049632210784920971491374075412750244140625;-0.07812530098165422487443976251597632654011249542236328125;-0.8390390626944819185695223495713435113430023193359375;-0.61228590615864764146181187243200838565826416015625;0.507430199299049977668119026930071413516998291015625;0.89382519717515040991173691509175114333629608154296875;0.412555605304473049788072103183367289602756500244140625]
      mask Display: e = 0.06;c1 = [120 150 230]/255;c2 = ([120 150 230]+0)/383;c3 = ([120 150 230]+128)/383;patch([0 1 1 0],[0 0 1 1],c1);patch([0 1 1 1-e 1-e 0+e],[0 0 1 1-e 0+e 0+e],c2);patch([0 0 1 1-e 0+e 0+e],[0 1 1 1-e 1-e 0+e],c3);plot([0 1 1 0 0],[0 0 1 1 0]);disp('weights');
  "IW{3,2}(2,:)'" : Constant  Value=[-0.255128163753369552324556934763677418231964111328125;-0.0222845815877221430090227016762582934461534023284912109375;-1.024418723681416398818555535399354994297027587890625;-0.430400373381310374920616368399350903928279876708984375;-0.68150150643284279627920341226854361593723297119140625;0.4289429464960881066559750252054072916507720947265625;0.1339849384100826601429190532144275493919849395751953125;0.55683718086817279857569928935845382511615753173828125;1.044230094168425271305977730662561953067779541015625;-0.4665516745296780509733025610330514609813690185546875;0.6721784492580977765641137011698447167873382568359375;0.5778969020539481693532479766872711479663848876953125;-0.2301774480410586354306445855399942956864833831787109375;-0.30193760778241240938513101355056278407573699951171875;0.212092888443818627930426146122044883668422698974609375;0.457286507189116953053797942629898898303508758544921875;0.3730464583610484385900463166763074696063995361328125;-0.83167293613590598244655893722665496170520782470703125;0.73221705999434016920446310905390419065952301025390625;-0.285218438923067696411095539588131941854953765869140625]
      mask Display: e = 0.06;c1 = [120 150 230]/255;c2 = ([120 150 230]+0)/383;c3 = ([120 150 230]+128)/383;patch([0 1 1 0],[0 0 1 1],c1);patch([0 1 1 1-e 1-e 0+e],[0 0 1 1-e 0+e 0+e],c2);patch([0 0 1 1-e 0+e 0+e],[0 1 1 1-e 1-e 0+e],c3);plot([0 1 1 0 0],[0 0 1 1 0]);disp('weights');
  Mux : Mux  Inputs=2
      mask Display: e = 0.06;c1 = [120 150 230]/255;c2 = ([120 150 230]+0)/383;c3 = ([120 150 230]+128)/383;patch([0 1 1 0],[0 0 1 1],c1);patch([0 1 1 1-e 1-e 0+e],[0 0 1 1-e 0+e 0+e],c2);patch([0 0 1 1-e 0+e 0+e],[0 1 1 1-e 1-e 0+e],c3);plot([0 1 1 0 0],[0 0 1 1 0]);disp('Mux');
  dotprod1 : neural/Weight Functions/dotprod
  dotprod2 : neural/Weight Functions/dotprod
  "lz{3,2}" : Outport
      mask Display: e = 0.06;c1 = [120 230 180]/255;c2 = ([120 230 180]+0)/(256+128);c3 = ([120 230 180]+128)/(256+128);patch([0 1 1 0],[0 0 1 1],c1);patch([0 1 1 1-e 1-e 0+e],[0 0 1 1-e 0+e 0+e],c2);patch([0 0 1 1-e 0+e 0+e],[0 1 1 1-e 1-e 0+e],c3);plot([0 1 1 0 0],[0 0 1 1 0]);
  wires:
  "IW{3,2}(1,:)'"/1 -> dotprod1/1
  "ad{3,2}"/1 -> dotprod1/2, dotprod2/2
  dotprod1/1 -> Mux/1
  "IW{3,2}(2,:)'"/1 -> dotprod2/1
  dotprod2/1 -> Mux/2
  Mux/1 -> "lz{3,2}"/1

== system MCVT_Integration/Sys2 - Structural System/Sys2_Structural_System/Fault Detection and Diagnosis (FDD) with Binary Classifer/Pattern Recognition Neural Network/Segment 4/Layer 1/Delays 1 ==
  "p{1}" : Inport  PortDimensions=2304
      mask Display: e = 0.06;c1 = [120 230 180]/255;c2 = ([120 230 180]+0)/(256+128);c3 = ([120 230 180]+128)/(256+128);patch([0 1 1 0],[0 0 1 1],c1);patch([0 1 1 1-e 1-e 0+e],[0 0 1 1-e 0+e 0+e],c2);patch([0 0 1 1-e 0+e 0+e],[0 1 1 1-e 1-e 0+e],c3);plot([0 1 1 0 0],[0 0 1 1 0]);
  mux : Mux  Inputs=1
      mask Display: e = 0.06;c1 = [120 150 230]/255;c2 = ([120 150 230]+0)/383;c3 = ([120 150 230]+128)/383;patch([0 1 1 0],[0 0 1 1],c1);patch([0 1 1 1-e 1-e 0+e],[0 0 1 1-e 0+e 0+e],c2);patch([0 0 1 1-e 0+e 0+e],[0 1 1 1-e 1-e 0+e],c3);plot([0 1 1 0 0],[0 0 1 1 0]);disp('Mux')
  "pd{1,1}" : Outport
      mask Display: e = 0.06;c1 = [120 230 180]/255;c2 = ([120 230 180]+0)/(256+128);c3 = ([120 230 180]+128)/(256+128);patch([0 1 1 0],[0 0 1 1],c1);patch([0 1 1 1-e 1-e 0+e],[0 0 1 1-e 0+e 0+e],c2);patch([0 0 1 1-e 0+e 0+e],[0 1 1 1-e 1-e 0+e],c3);plot([0 1 1 0 0],[0 0 1 1 0]);
  wires:
  "p{1}"/1 -> mux/1
  mux/1 -> "pd{1,1}"/1
